simulink model "simulation_environment_thesis"  (R2024b)
settings: Solver=VariableStepAuto  StartTime=0.0  StopTime=3200  RelTol=1e-3
values from neighbouring files (symbols this model uses):
  A = [1, dt; 0, 1]    [estimate_and_control_fully_actuated_with_current.m]
  A1 = [1, 0, 0, 0, dt, 0 ... (first row only)    [estimate_and_control_fully_actuated_with_current.m]
  alpha = 10    [estimate_and_control_fully_actuated_with_current.m]
  alpha_xy_1 = 1    [estimate_and_control_fully_actuated_with_current.m]
  alpha_z_1 = 5    [estimate_and_control_fully_actuated_with_current.m]
  Az1 = [1, dt ... (first row only)    [estimate_and_control_fully_actuated_with_current.m]
  B = [dt; 0]    [estimate_and_control_fully_actuated_with_current.m]
  B1 = [dt, 0 ... (first row only)    [estimate_and_control_fully_actuated_with_current.m]
  Bz1 = [dt ... (first row only)    [estimate_and_control_fully_actuated_with_current.m]
  C1 = [1, 0, 0, 0, 0, 0 ... (first row only)    [estimate_and_control_fully_actuated_with_current.m]
  circumference_x = min_dist_from_ds*cos(theta)    [estimate_and_control_fully_actuated_with_current.m]
  circumference_y = min_dist_from_ds*sin(theta)    [estimate_and_control_fully_actuated_with_current.m]
  const_current = [-r; -s; 0; 0]    [estimate_and_control_fully_actuated_with_current.m]
  csi_psi_1 = 0.0003    [estimate_and_control_fully_actuated_with_current.m]
  csi_r_1 = 0.0003    [estimate_and_control_fully_actuated_with_current.m]
  csi_vcx_1 = 0.0003    [estimate_and_control_fully_actuated_with_current.m]
  csi_vcy_1 = 0.0003    [estimate_and_control_fully_actuated_with_current.m]
  csi_vcz_1 = 0.005    [estimate_and_control_fully_actuated_with_current.m]
  csi_x_1 = 0.003    [estimate_and_control_fully_actuated_with_current.m]
  csi_y_1 = 0.003    [estimate_and_control_fully_actuated_with_current.m]
  csi_z_1 = 0.005    [estimate_and_control_fully_actuated_with_current.m]
  Cz1 = [1, 0]    [estimate_and_control_fully_actuated_with_current.m]
  d = 1    [estimate_and_control_fully_actuated_with_current.m]
  delta_FOV = pi/7    (= 0.448798950513)    [estimate_and_control_fully_actuated_with_current.m]
  desired_speed = speed_0    [estimate_and_control_fully_actuated_with_current.m]
  dt = 1/Tsim    (= 0.005)    [estimate_and_control_fully_actuated_with_current.m]
  eta1 = deg2rad(0.5)    [estimate_and_control_fully_actuated_with_current.m]
  eta2 = deg2rad(0.5)    [estimate_and_control_fully_actuated_with_current.m]
  eta_psi_1 = 0.003    [estimate_and_control_fully_actuated_with_current.m]
  eta_x_1 = 0.03    [estimate_and_control_fully_actuated_with_current.m]
  eta_y_1 = 0.03    [estimate_and_control_fully_actuated_with_current.m]
  eta_z_1 = 0.005    [estimate_and_control_fully_actuated_with_current.m]
  G = dt*eye(2)    [estimate_and_control_fully_actuated_with_current.m]
  G1 = dt*eye(6)    [estimate_and_control_fully_actuated_with_current.m]
  Gz1 = dt*eye(2)    [estimate_and_control_fully_actuated_with_current.m]
  left_reference = L_x*[cos(NED_psi_ds); sin(NED_psi_ds); 0] + L_y_l*[-sin(NED_psi_ds); cos(NED_psi_ds); 0]    [estimate_and_control_fully_actuated_with_current.m]
  max_dist_from_ship = 60    [estimate_and_control_fully_actuated_with_current.m]
  min_dist_from_ds = 45    [estimate_and_control_fully_actuated_with_current.m]
  mu = -5    [estimate_and_control_fully_actuated_with_current.m]
  NED_psi_ds = -pi/2    (= -1.57079632679)    [estimate_and_control_fully_actuated_with_current.m]
  p_b1 = [-90; 90; 5]    [estimate_and_control_fully_actuated_with_current.m]
  position_t_0 = [0; 0; 0; 0]    [estimate_and_control_fully_actuated_with_current.m]
  psi_P = linspace(0,2*pi,360)'    [estimate_and_control_fully_actuated_with_current.m]
  Q = [csi1_kf^2, 0 ... (first row only)    [estimate_and_control_fully_actuated_with_current.m]
  Q1 = [csi_x_1_kf^2, 0, 0, 0, 0, 0 ... (first row only)    [estimate_and_control_fully_actuated_with_current.m]
  Qz1 = [csi_z_1_kf^2, 0 ... (first row only)    [estimate_and_control_fully_actuated_with_current.m]
  R = [eta1_kf^2, 0 ... (first row only)    [estimate_and_control_fully_actuated_with_current.m]
  R1 = [eta_x_1_kf^2, 0, 0 ... (first row only)    [estimate_and_control_fully_actuated_with_current.m]
  right_reference = L_x*[cos(NED_psi_ds); sin(NED_psi_ds); 0] + L_y_r*[sin(NED_psi_ds); -cos(NED_psi_ds); 0]    [estimate_and_control_fully_actuated_with_current.m]
  Rz1 = eta_z_1_kf^2    (= 1e-06)    [estimate_and_control_fully_actuated_with_current.m]
  speed_0 = [0.1, 0.1, 0, 0]'    [estimate_and_control_fully_actuated_with_current.m]
  tau_heave_sat = 30    [estimate_and_control_fully_actuated_with_current.m]
  tau_surge_sat = 10    [estimate_and_control_fully_actuated_with_current.m]
  tau_sway_sat = 30    [estimate_and_control_fully_actuated_with_current.m]
  tau_yaw_sat = 0.1    [estimate_and_control_fully_actuated_with_current.m]
  var_vm_x = 0.001    [estimate_and_control_fully_actuated_with_current.m]
  var_vm_y = 0.001    [estimate_and_control_fully_actuated_with_current.m]
  var_vm_z = 0.001    [estimate_and_control_fully_actuated_with_current.m]
undefined symbols (not defined in the file or in its folder): A2, alpha2, B2, csi1_2, csi2_2, csi3_2, csi4_2, csi5_2, csi6_2, csi7_2, csi8_2, eta1_2, eta2_2, eta3_2, eta4_2, eta5_2, eta6_2, G2, Q2, R2
libraries: simulink x60, simulink_extras x5
(part 6 of 16)

== system simulation_environment_thesis/PHASE 3&4.21/PHASE  3 ==
  note: ds_psi_auv
  note: ok, trasformazione coerente
  note: ok cominciano esattamente dalla flag
  note: physical data to be sent to
      | the range-measurement simulator
  ship_psi_auv : Inport
  ship_p_auv : Inport  Port=2
  ship_p_ds : Inport  Port=3
  Enable : EnablePort
  CONTROLLER4 : Subsystem
  Constant : Constant  Value=0
  Constant9 : Constant  Value=desired_speed
  Data Store Read : DataStoreRead  DataStoreName=x_hat_23_2  IndexOptions=Index vector (dialog)  Indices=1  OutputSizes=1
  Data Store Read1 : DataStoreRead  DataStoreName=P0_23_2  IndexOptions=Index vector (dialog)  Indices=1  OutputSizes=1
  Data Store Write : DataStoreWrite  DataStoreName=x_hat_34_2  IndexOptions=Index vector (dialog)  Indices=1  OutputSizes=1
  Data Store Write1 : DataStoreWrite  DataStoreName=P0_34_2  IndexOptions=Index vector (dialog)  Indices=1  OutputSizes=1
  MATLAB Function : MATLAB Function  TreatAsAtomicUnit=on
  MATLAB Function1 : MATLAB Function  TreatAsAtomicUnit=on
  Scope1 : Scope
  Selector1 : Selector  InputPortWidth=4  IndexOptions=Index vector (dialog)  Indices=[1 2 3]  OutputSizes=1
  Selector2 : Selector  InputPortWidth=3  IndexOptions=Index vector (dialog)  Indices=[3]  OutputSizes=1
  Selector3 : Selector  InputPortWidth=3  IndexOptions=Index vector (dialog)  Indices=[1 2]  OutputSizes=1
  Selector4 : Selector  InputPortWidth=8  IndexOptions=Index vector (dialog)  Indices=[1 2 7 8 4 5]  OutputSizes=1
  Selector5 : Selector  InputPortWidth=8  IndexOptions=Index vector (dialog)  Indices=[3 6]  OutputSizes=1
  Sum : Sum  Inputs=|+-
  TAU : Scope
  To Workspace : ToWorkspace  VariableName=switchposition23  SampleTime=-1
  XY PATH GUIDANCE : Subsystem
  dynamic + kinematic models1 : Subsystem
  "p, v_c, psi, r (3D) estimate, from RANGE, v_c, PSI measurement" : Subsystem
  v error (robot frame)5 : Scope
  yaw_err3 : Scope
  z_err : Scope
  speed body : Outport
  s_p_auv : Outport  Port=2
  s_psi_auv : Outport  Port=3
  ds_p_auv : Outport  Port=4
  state_est_plane : Outport  Port=5
  state_est_z : Outport  Port=6
  "z_err " : Outport  Port=7
  error speed : Outport  Port=8
  yaw_error : Outport  Port=9
  wires:
  CONTROLLER4/1(tau) -> dynamic + kinematic models1/1(tau), TAU/1
  Constant9/1 -> dynamic + kinematic models1/2(ref speed), CONTROLLER4/3(speed_0), XY PATH GUIDANCE/1(speed)
  dynamic + kinematic models1/1(error speed) -> CONTROLLER4/1(v_err), error speed/1, v error (robot frame)5/1
  MATLAB Function/1(ds_p_auv) -> dynamic + kinematic models1/5(ds_p_auv), Scope1/1
  ship_psi_auv/1 -> dynamic + kinematic models1/3(last_yaw__from_ship)
  ship_p_ds/1 -> MATLAB Function1/2(ship_p_ds), MATLAB Function/2(ship_p_ds)
  dynamic + kinematic models1/3(p) -> Selector1/1
  MATLAB Function1/1(ship_p_auv) -> s_p_auv/1
  dynamic + kinematic models1/4(yaw) -> "p, v_c, psi, r (3D) estimate, from RANGE, v_c, PSI measurement"/3(psi), s_psi_auv/1
  dynamic + kinematic models1/5(body speed) -> speed body/1
  Sum/1 -> CONTROLLER4/4(z_err), "z_err "/1, z_err/1
  Constant/1 -> Sum/1
  XY PATH GUIDANCE/1(yaw_error) -> CONTROLLER4/2(psi_err), yaw_err3/1, yaw_error/1
  Selector2/1 -> Sum/2
  "p, v_c, psi, r (3D) estimate, from RANGE, v_c, PSI measurement"/1(xyz_est) -> Selector2/1, Selector3/1
  "p, v_c, psi, r (3D) estimate, from RANGE, v_c, PSI measurement"/2(psi_est) -> dynamic + kinematic models1/4(yaw_est), XY PATH GUIDANCE/3(yaw_est)
  "p, v_c, psi, r (3D) estimate, from RANGE, v_c, PSI measurement"/3(v_c_xyz) -> XY PATH GUIDANCE/4(v_c)
  Selector3/1 -> XY PATH GUIDANCE/2(eta)
  dynamic + kinematic models1/2(v_tot ds) -> "p, v_c, psi, r (3D) estimate, from RANGE, v_c, PSI measurement"/2(inert_vel)
  Selector1/1 -> MATLAB Function1/1(ds_p_auv), ds_p_auv/1, "p, v_c, psi, r (3D) estimate, from RANGE, v_c, PSI measurement"/1(p)
  Data Store Read/1 -> "p, v_c, psi, r (3D) estimate, from RANGE, v_c, PSI measurement"/4(x_0_hat)
  Data Store Read1/1 -> "p, v_c, psi, r (3D) estimate, from RANGE, v_c, PSI measurement"/5(P0)
  "p, v_c, psi, r (3D) estimate, from RANGE, v_c, PSI measurement"/5(P0_out) -> Data Store Write1/1
  ship_p_auv/1 -> MATLAB Function/1(ship_p_auv), To Workspace/1
  Selector5/1 -> state_est_z/1
  "p, v_c, psi, r (3D) estimate, from RANGE, v_c, PSI measurement"/4(state_est) -> Selector5/1, Selector4/1, Data Store Write/1
  Selector4/1 -> state_est_plane/1

== system simulation_environment_thesis/PHASE 3&4.21/PHASE  4 ==
  note: ds_psi_auv
  note: ok, trasformazione coerente
  note: ok cominciano esattamente dalla flag
  note: i know the beacon is my new
      | reference frame, so (0,0,0)
  note: physical data to be sent to
      | the range-measurement simulator
  ship_psi_auv : Inport
  ship_p_auv : Inport  Port=2
  ship_p_ds : Inport  Port=3
  direct_to_4 : Inport  Port=4
  Enable : EnablePort
  CONTROLLER4 : Subsystem
  Constant : Constant  Value=0
  Constant3 : Constant  Value=norm(speed_0)
  Constant5 : Constant  Value=[0; 0]  VectorParams1D=off
  Constant7 : Constant  Value=NED_psi_ds + pi
  Data Store Read : DataStoreRead  DataStoreName=x_hat_23_2  IndexOptions=Index vector (dialog)  Indices=1  OutputSizes=1
  Data Store Read1 : DataStoreRead  DataStoreName=x_hat_34_2  IndexOptions=Index vector (dialog)  Indices=1  OutputSizes=1
  Data Store Read2 : DataStoreRead  DataStoreName=P0_23_2  IndexOptions=Index vector (dialog)  Indices=1  OutputSizes=1
  Data Store Read3 : DataStoreRead  DataStoreName=P0_34_2  IndexOptions=Index vector (dialog)  Indices=1  OutputSizes=1
  MATLAB Function : MATLAB Function  TreatAsAtomicUnit=on
  MATLAB Function1 : MATLAB Function  TreatAsAtomicUnit=on
  MATLAB Function11 : MATLAB Function  TreatAsAtomicUnit=on
  MATLAB Function6 : MATLAB Function  TreatAsAtomicUnit=on
  MATLAB Function7 : MATLAB Function  TreatAsAtomicUnit=on
  MATLAB Function8 : MATLAB Function  Commented=on  TreatAsAtomicUnit=on
  MATLAB Function9 : MATLAB Function  TreatAsAtomicUnit=on
  Memory : Memory
  Scope{1,4,6,8..9} (5 alike) : Scope
  Selector1 : Selector  InputPortWidth=4  IndexOptions=Index vector (dialog)  Indices=[1 2 3]  OutputSizes=1
  Selector3 : Selector  InputPortWidth=3  IndexOptions=Index vector (dialog)  Indices=[3]  OutputSizes=1
  Selector4 : Selector  InputPortWidth=3  IndexOptions=Index vector (dialog)  Indices=[1 2]  OutputSizes=1
  Selector5 : Selector  InputPortWidth=3  IndexOptions=Index vector (dialog)  Indices=[1 2]  OutputSizes=1
  Selector6 : Selector  InputPortWidth=8  IndexOptions=Index vector (dialog)  Indices=[1 2 7 8 4 5]  OutputSizes=1
  Selector7 : Selector  InputPortWidth=8  IndexOptions=Index vector (dialog)  Indices=[3 6]  OutputSizes=1
  Stop Simulation : Stop
  Subsystem : Subsystem
  Sum : Sum  Inputs=|+-
  Sum4 : Sum  Inputs=|+-
  Switch3 : Switch  Criteria=u2 > Threshold  SaturateOnIntegerOverflow=off  InputSameDT=off
  Switch4 : Switch  Criteria=u2 > Threshold  SaturateOnIntegerOverflow=off  InputSameDT=off
  TAU : Scope
  To Workspace : ToWorkspace  VariableName=switchposition34  SampleTime=-1
  To Workspace1 : ToWorkspace  VariableName=docked  SampleTime=-1
  Unit Delay : UnitDelay  SampleTime=-1  HasFrameUpgradeWarning=on
  dynamic + kinematic models1 : Subsystem
  "p, v_c, psi, r (3D) estimate, from RANGE, v_c, PSI measurement" : Subsystem
  "positon and current estimate, XY PLANE from X, Y, psi measurement" : Subsystem  Commented=on
  v error (robot frame)5 : Scope
  z_err : Scope
  speed body : Outport
  s_p_auv : Outport  Port=2
  s_psi_auv : Outport  Port=3
  new_speed_desired : Outport  Port=4
  docked : Outport  Port=5
  state_est_plane : Outport  Port=6
  state_est_z : Outport  Port=7
  ds_p_auv_4 : Outport  Port=8
  z_ err : Outport  Port=9
  angle : Outport  Port=10
  error speed : Outport  Port=11
  wires:
  CONTROLLER4/1(tau) -> dynamic + kinematic models1/1(tau), TAU/1
  dynamic + kinematic models1/1(error speed) -> error speed/1, CONTROLLER4/1(v_err), v error (robot frame)5/1
  MATLAB Function/1(ds_p_auv) -> Scope1/1, dynamic + kinematic models1/5(ds_p_auv)
  ship_psi_auv/1 -> dynamic + kinematic models1/3(last_yaw__from_ship)
  ship_p_ds/1 -> MATLAB Function/2(ship_p_ds), MATLAB Function1/2(ship_p_ds)
  dynamic + kinematic models1/3(p) -> Selector1/1
  MATLAB Function1/1(ship_p_auv) -> s_p_auv/1
  dynamic + kinematic models1/4(yaw) -> s_psi_auv/1, "p, v_c, psi, r (3D) estimate, from RANGE, v_c, PSI measurement"/3(psi)
  dynamic + kinematic models1/5(body speed) -> speed body/1
  Selector3/1 -> Sum/2
  Sum/1 -> z_ err/1, CONTROLLER4/4(z_err), z_err/1
  Constant/1 -> Sum/1
  dynamic + kinematic models1/2(v_tot ds) -> "p, v_c, psi, r (3D) estimate, from RANGE, v_c, PSI measurement"/2(inert_vel)
  Sum4/1 -> MATLAB Function6/1("angle ")
  MATLAB Function6/1(angle) -> angle/1, Scope9/1, CONTROLLER4/2(psi_err)
  Unit Delay/1 -> CONTROLLER4/3(speed_0), dynamic + kinematic models1/2(ref speed)
  "p, v_c, psi, r (3D) estimate, from RANGE, v_c, PSI measurement"/2(psi_est) -> Sum4/2, dynamic + kinematic models1/4(yaw_est)
  Selector1/1 -> "p, v_c, psi, r (3D) estimate, from RANGE, v_c, PSI measurement"/1(p), MATLAB Function1/1(ds_p_auv), ds_p_auv_4/1
  Switch3/1 -> "p, v_c, psi, r (3D) estimate, from RANGE, v_c, PSI measurement"/4(x_0_hat)
  Data Store Read/1 -> Switch3/1
  Data Store Read1/1 -> Switch3/3
  Data Store Read2/1 -> Switch4/1
  Data Store Read3/1 -> Switch4/3
  direct_to_4/1 -> Switch4/2, Switch3/2
  Switch4/1 -> "p, v_c, psi, r (3D) estimate, from RANGE, v_c, PSI measurement"/5(P0)
  ship_p_auv/1 -> To Workspace/1, MATLAB Function/1(ship_p_auv)
  Subsystem/1(w) -> MATLAB Function7/3(w)
  MATLAB Function11/1(angle) -> Subsystem/1(NED_psi_dock), MATLAB Function7/4(NED_psi_dock)
  "p, v_c, psi, r (3D) estimate, from RANGE, v_c, PSI measurement"/3(v_c_xyz) -> Selector5/1
  MATLAB Function7/1(v_blend) -> Scope4/1, Unit Delay/1, new_speed_desired/1, Scope6/1
  "p, v_c, psi, r (3D) estimate, from RANGE, v_c, PSI measurement"/1(xyz_est) -> Selector4/1, Subsystem/3(eta), Selector3/1
  Selector4/1 -> MATLAB Function9/1(ds_p_auv), Subsystem/2(x_y)
  Subsystem/2(speed_desired_circ) -> MATLAB Function7/2(v_circ)
  MATLAB Function9/2(range) -> Scope8/1
  Constant7/1 -> Sum4/1, MATLAB Function11/1("angle ")
  Memory/1 -> MATLAB Function9/3("docked ")
  Subsystem/4(speed_desired_lin) -> MATLAB Function7/1(v_line)
  Constant5/1 -> MATLAB Function9/2(ds_p_ds)
  Constant3/1 -> Subsystem/5(v_tot_des)
  Subsystem/3(d) -> MATLAB Function7/5(d)
  Selector5/1 -> Subsystem/4(v_c)
  "p, v_c, psi, r (3D) estimate, from RANGE, v_c, PSI measurement"/4(state_est) -> Selector6/1, Selector7/1
  Selector7/1 -> state_est_z/1
  Selector6/1 -> state_est_plane/1
  MATLAB Function9/1(docked) -> To Workspace1/1, docked/1, Memory/1, Stop Simulation/1

== system simulation_environment_thesis/PHASE 3&4.21/Subsystem3 ==
  num : Inport
  Enable : EnablePort
  MATLAB Function7 : MATLAB Function  TreatAsAtomicUnit=on
  out : Outport
  wires:
  num/1 -> MATLAB Function7/1(num)
  MATLAB Function7/1(out) -> out/1

== system simulation_environment_thesis/PHASE 3&4.21/end phase 3 ==
  note: the DS is in the origin
      | of the DS frame
  ds_p_auv : Inport
  Enable : EnablePort
  # of pings : MATLAB Function  TreatAsAtomicUnit=on
  Constant : Constant  Value=NED_psi_ds
  Constant13 : Constant  Value=[0; 0]  VectorParams1D=off
  Constant8 : Constant  Value=delta_FOV
  Scope3 : Scope
  num_of_pings : Outport
  wires:
  Constant8/1 -> # of pings/2(delta)
  ds_p_auv/1 -> # of pings/4(ds_p_auv)
  # of pings/1(num_of_pings) -> num_of_pings/1, Scope3/1
  Constant13/1 -> # of pings/3(ds_p_ds)
  Constant/1 -> # of pings/1(alpha_ds)

== system simulation_environment_thesis/PHASE 3&4.21/logic to recognize where i am, based on the # of pings ==
  note: se out è 1 --> phase 4
      | se out è 0 --> phase 3
  num : Inport
  MATLAB Function6 : MATLAB Function  TreatAsAtomicUnit=on
  out : Outport
  wires:
  MATLAB Function6/1(out) -> out/1
  num/1 -> MATLAB Function6/1(num)

== system simulation_environment_thesis/PHASE 3&4.21/simulator of pings from sensor ==
  note: the DS is in the origin
      | of the DS frame
  ds_p_auv : Inport
  stop_flag : Inport  Port=2
  # of pings : MATLAB Function  TreatAsAtomicUnit=on
  Constant : Constant  Value=NED_psi_ds
  Constant13 : Constant  Value=[0; 0]  VectorParams1D=off
  Constant8 : Constant  Value=delta_FOV
  Scope3 : Scope
  # OF PINGS : Outport
  wires:
  Constant/1 -> # of pings/1(alpha_ds)
  # of pings/1(num_of_pings) -> Scope3/1, # OF PINGS/1
  Constant13/1 -> # of pings/3(ds_p_ds)
  Constant8/1 -> # of pings/2(delta)
  ds_p_auv/1 -> # of pings/4(ds_p_auv)
  stop_flag/1 -> # of pings/5(stop_flag)

== system simulation_environment_thesis/PHASE 3&4.22/PHASE  3 ==
  note: ds_psi_auv
  note: ok, trasformazione coerente
  note: ok cominciano esattamente dalla flag
  note: physical data to be sent to
      | the range-measurement simulator
  ship_psi_auv : Inport
  ship_p_auv : Inport  Port=2
  ship_p_ds : Inport  Port=3
  Enable : EnablePort
  CONTROLLER4 : Subsystem
  Constant : Constant  Value=0
  Constant9 : Constant  Value=desired_speed
  Data Store Read : DataStoreRead  DataStoreName=x_hat_23_3  IndexOptions=Index vector (dialog)  Indices=1  OutputSizes=1
  Data Store Read1 : DataStoreRead  DataStoreName=P0_23_3  IndexOptions=Index vector (dialog)  Indices=1  OutputSizes=1
  Data Store Write : DataStoreWrite  DataStoreName=x_hat_34_3  IndexOptions=Index vector (dialog)  Indices=1  OutputSizes=1
  Data Store Write1 : DataStoreWrite  DataStoreName=P0_34_3  IndexOptions=Index vector (dialog)  Indices=1  OutputSizes=1
  MATLAB Function : MATLAB Function  TreatAsAtomicUnit=on
  MATLAB Function1 : MATLAB Function  TreatAsAtomicUnit=on
  Scope1 : Scope
  Selector1 : Selector  InputPortWidth=4  IndexOptions=Index vector (dialog)  Indices=[1 2 3]  OutputSizes=1
  Selector2 : Selector  InputPortWidth=3  IndexOptions=Index vector (dialog)  Indices=[3]  OutputSizes=1
  Selector3 : Selector  InputPortWidth=3  IndexOptions=Index vector (dialog)  Indices=[1 2]  OutputSizes=1
  Selector4 : Selector  InputPortWidth=8  IndexOptions=Index vector (dialog)  Indices=[1 2 7 8 4 5]  OutputSizes=1
  Selector5 : Selector  InputPortWidth=8  IndexOptions=Index vector (dialog)  Indices=[3 6]  OutputSizes=1
  Sum : Sum  Inputs=|+-
  TAU : Scope
  To Workspace : ToWorkspace  VariableName=switchposition23  SampleTime=-1
  XY PATH GUIDANCE : Subsystem
  dynamic + kinematic models1 : Subsystem
  "p, v_c, psi, r (3D) estimate, from RANGE, v_c, PSI measurement" : Subsystem
  v error (robot frame)5 : Scope
  yaw_err3 : Scope
  z_err : Scope
  speed body : Outport
  s_p_auv : Outport  Port=2
  s_psi_auv : Outport  Port=3
  ds_p_auv : Outport  Port=4
  state_est_plane : Outport  Port=5
  state_est_z : Outport  Port=6
  error speed : Outport  Port=7
  yaw_error : Outport  Port=8
  z_ err : Outport  Port=9
  wires:
  Constant9/1 -> dynamic + kinematic models1/2(ref speed), CONTROLLER4/3(speed_0), XY PATH GUIDANCE/1(speed)
  CONTROLLER4/1(tau) -> dynamic + kinematic models1/1(tau), TAU/1
  dynamic + kinematic models1/1(error speed) -> error speed/1, CONTROLLER4/1(v_err), v error (robot frame)5/1
  MATLAB Function/1(ds_p_auv) -> dynamic + kinematic models1/5(ds_p_auv), Scope1/1
  ship_psi_auv/1 -> dynamic + kinematic models1/3(last_yaw__from_ship)
  ship_p_ds/1 -> MATLAB Function1/2(ship_p_ds), MATLAB Function/2(ship_p_ds)
  dynamic + kinematic models1/4(p) -> Selector1/1
  MATLAB Function1/1(ship_p_auv) -> s_p_auv/1
  dynamic + kinematic models1/5(yaw) -> s_psi_auv/1, "p, v_c, psi, r (3D) estimate, from RANGE, v_c, PSI measurement"/3(psi)
  dynamic + kinematic models1/3(body speed) -> speed body/1
  Sum/1 -> CONTROLLER4/4(z_err), z_ err/1, z_err/1
  Constant/1 -> Sum/1
  XY PATH GUIDANCE/1(yaw_error) -> CONTROLLER4/2(psi_err), yaw_err3/1, yaw_error/1
  Selector2/1 -> Sum/2
  "p, v_c, psi, r (3D) estimate, from RANGE, v_c, PSI measurement"/1(xyz_est) -> Selector2/1, Selector3/1
  "p, v_c, psi, r (3D) estimate, from RANGE, v_c, PSI measurement"/2(psi_est) -> dynamic + kinematic models1/4(yaw_est), XY PATH GUIDANCE/3(yaw_est)
  "p, v_c, psi, r (3D) estimate, from RANGE, v_c, PSI measurement"/3(v_c_xyz) -> XY PATH GUIDANCE/4(v_c)
  Selector3/1 -> XY PATH GUIDANCE/2(eta)
  dynamic + kinematic models1/2(v_tot ds) -> "p, v_c, psi, r (3D) estimate, from RANGE, v_c, PSI measurement"/2(inert_vel)
  Selector1/1 -> MATLAB Function1/1(ds_p_auv), ds_p_auv/1, "p, v_c, psi, r (3D) estimate, from RANGE, v_c, PSI measurement"/1(p)
  Data Store Read/1 -> "p, v_c, psi, r (3D) estimate, from RANGE, v_c, PSI measurement"/4(x_0_hat)
  Data Store Read1/1 -> "p, v_c, psi, r (3D) estimate, from RANGE, v_c, PSI measurement"/5(P0)
  "p, v_c, psi, r (3D) estimate, from RANGE, v_c, PSI measurement"/5(P0_out) -> Data Store Write1/1
  ship_p_auv/1 -> MATLAB Function/1(ship_p_auv), To Workspace/1
  Selector5/1 -> state_est_z/1
  Selector4/1 -> state_est_plane/1
  "p, v_c, psi, r (3D) estimate, from RANGE, v_c, PSI measurement"/4(state_est) -> Selector4/1, Selector5/1, Data Store Write/1

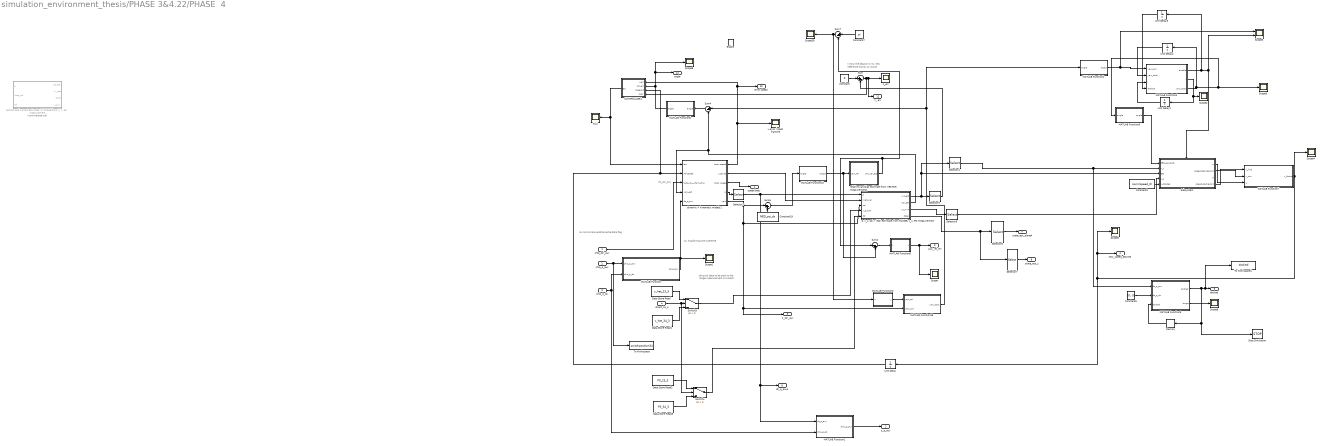
== system simulation_environment_thesis/PHASE 3&4.22/PHASE  4 ==
  note: ds_psi_auv
  note: ok, trasformazione coerente
  note: ok cominciano esattamente dalla flag
  note: i know the beacon is my new
      | reference frame, so (0,0,0)
  note: physical data to be sent to
      | the range-measurement simulator
  ship_psi_auv : Inport
  ship_p_auv : Inport  Port=2
  ship_p_ds : Inport  Port=3
  direct_to_4 : Inport  Port=4
  Enable : EnablePort
  CONTROLLER4 : Subsystem
  Constant : Constant  Value=0
  Constant19 : Constant  Value=NED_psi_ds
  Constant3 : Constant  Value=norm(speed_0)
  Constant5 : Constant  Value=[0; 0]  VectorParams1D=off
  Constant7 : Constant  Value=pi
  Data Store Read : DataStoreRead  DataStoreName=x_hat_23_3  IndexOptions=Index vector (dialog)  Indices=1  OutputSizes=1
  Data Store Read1 : DataStoreRead  DataStoreName=x_hat_34_3  IndexOptions=Index vector (dialog)  Indices=1  OutputSizes=1
  Data Store Read2 : DataStoreRead  DataStoreName=P0_23_3  IndexOptions=Index vector (dialog)  Indices=1  OutputSizes=1
  Data Store Read3 : DataStoreRead  DataStoreName=P0_34_3  IndexOptions=Index vector (dialog)  Indices=1  OutputSizes=1
  MATLAB Function : MATLAB Function  TreatAsAtomicUnit=on
  MATLAB Function1 : MATLAB Function  TreatAsAtomicUnit=on
  MATLAB Function10 : MATLAB Function  TreatAsAtomicUnit=on
  MATLAB Function18 : MATLAB Function  TreatAsAtomicUnit=on
  MATLAB Function2 : MATLAB Function  TreatAsAtomicUnit=on
  MATLAB Function3 : MATLAB Function  TreatAsAtomicUnit=on
  MATLAB Function4 : MATLAB Function  TreatAsAtomicUnit=on
  MATLAB Function5 : MATLAB Function  TreatAsAtomicUnit=on
  MATLAB Function6 : MATLAB Function  TreatAsAtomicUnit=on
  MATLAB Function7 : MATLAB Function  TreatAsAtomicUnit=on
  MATLAB Function8 : MATLAB Function  TreatAsAtomicUnit=on
  MATLAB Function9 : MATLAB Function  TreatAsAtomicUnit=on
  Memory : Memory
  Scope{,1..4,6,8..9,29} (9 alike) : Scope
  Scope5 : Scope  NumInputPorts=2
  Selector1 : Selector  InputPortWidth=4  IndexOptions=Index vector (dialog)  Indices=[1 2 3]  OutputSizes=1
  Selector3 : Selector  InputPortWidth=3  IndexOptions=Index vector (dialog)  Indices=[3]  OutputSizes=1
  Selector4 : Selector  InputPortWidth=3  IndexOptions=Index vector (dialog)  Indices=[1 2]  OutputSizes=1
  Selector5 : Selector  InputPortWidth=3  IndexOptions=Index vector (dialog)  Indices=[1 2]  OutputSizes=1
  Selector6 : Selector  InputPortWidth=8  IndexOptions=Index vector (dialog)  Indices=[1 2 7 8 4 5]  OutputSizes=1
  Selector7 : Selector  InputPortWidth=8  IndexOptions=Index vector (dialog)  Indices=[3 6]  OutputSizes=1
  Stop Simulation : Stop
  Subsystem : Subsystem
  Sum : Sum  Inputs=|+-
  Sum2 : Sum  Inputs=|-+
  Sum4 : Sum  Inputs=|+-
  Sum6 : Sum  Inputs=|-+
  Sum7 : Sum  Inputs=|+-
  Switch3 : Switch  Criteria=u2 > Threshold  SaturateOnIntegerOverflow=off  InputSameDT=off
  Switch4 : Switch  Criteria=u2 > Threshold  SaturateOnIntegerOverflow=off  InputSameDT=off
  TAU : Scope
  To Workspace : ToWorkspace  VariableName=switchposition34  SampleTime=-1
  To Workspace1 : ToWorkspace  VariableName=docked  SampleTime=-1
  Unit Delay{,1..3} (4 alike) : UnitDelay  SampleTime=-1  HasFrameUpgradeWarning=on
  dynamic + kinematic models1 : Subsystem
  "p, v_c, psi, r (3D) estimate, from RANGE, v_c, PSI measurement" : Subsystem
  "positon and current estimate, XY PLANE from X, Y, psi measurement" : Subsystem  Commented=on
  relative yaw/bias estimate from VECTOR measurement1 : Subsystem
  v error (robot frame)5 : Scope
  z_err : Scope
  speed body : Outport
  s_p_auv : Outport  Port=2
  s_psi_auv : Outport  Port=3
  new_speed_desired : Outport  Port=4
  docked : Outport  Port=5
  ds_p_auv4 : Outport  Port=6
  state_est_plane4 : Outport  Port=7
  state_est_z : Outport  Port=8
  yaw_rel_err : Outport  Port=9
  error speed : Outport  Port=10
  angle : Outport  Port=11
  "z_ err " : Outport  Port=12
  wires:
  CONTROLLER4/1(tau) -> dynamic + kinematic models1/1(tau), TAU/1
  dynamic + kinematic models1/1(error speed) -> error speed/1, CONTROLLER4/1(v_err), v error (robot frame)5/1
  MATLAB Function/1(ds_p_auv) -> dynamic + kinematic models1/5(ds_p_auv), Scope1/1
  ship_psi_auv/1 -> dynamic + kinematic models1/3(last_yaw__from_ship)
  ship_p_ds/1 -> MATLAB Function/2(ship_p_ds), MATLAB Function1/2(ship_p_ds)
  dynamic + kinematic models1/4(p) -> Selector1/1
  MATLAB Function1/1(ship_p_auv) -> s_p_auv/1
  dynamic + kinematic models1/5(yaw) -> MATLAB Function18/2(yaw_curr), s_psi_auv/1, "p, v_c, psi, r (3D) estimate, from RANGE, v_c, PSI measurement"/3(psi), Sum6/1
  dynamic + kinematic models1/3(body speed) -> speed body/1
  Selector3/1 -> Sum/2
  Sum/1 -> CONTROLLER4/4(z_err), "z_ err "/1, z_err/1
  Constant/1 -> Sum/1
  dynamic + kinematic models1/2(v_tot ds) -> "p, v_c, psi, r (3D) estimate, from RANGE, v_c, PSI measurement"/2(inert_vel)
  "p, v_c, psi, r (3D) estimate, from RANGE, v_c, PSI measurement"/3(v_c_xyz) -> Selector5/1
  Sum4/1 -> MATLAB Function6/1("angle ")
  MATLAB Function6/1(angle) -> angle/1, Scope9/1, CONTROLLER4/2(psi_err)
  "p, v_c, psi, r (3D) estimate, from RANGE, v_c, PSI measurement"/2(psi_est) -> dynamic + kinematic models1/4(yaw_est), Sum4/2
  Selector1/1 -> "p, v_c, psi, r (3D) estimate, from RANGE, v_c, PSI measurement"/1(p), ds_p_auv4/1, MATLAB Function1/1(ds_p_auv)
  Switch3/1 -> "p, v_c, psi, r (3D) estimate, from RANGE, v_c, PSI measurement"/4(x_0_hat)
  Data Store Read/1 -> Switch3/1
  Data Store Read1/1 -> Switch3/3
  Data Store Read2/1 -> Switch4/1
  Data Store Read3/1 -> Switch4/3
  direct_to_4/1 -> Switch4/2, Switch3/2
  Switch4/1 -> "p, v_c, psi, r (3D) estimate, from RANGE, v_c, PSI measurement"/5(P0)
  Constant19/1 -> Sum6/2
  Sum6/1 -> MATLAB Function10/1("angle ")
  Constant7/1 -> Sum7/1
  relative yaw/bias estimate from VECTOR measurement1/1(yaw_rel_est) -> Sum2/1, Sum7/2
  Sum7/1 -> Scope29/1, MATLAB Function3/1(u)
  Unit Delay/1 -> dynamic + kinematic models1/2(ref speed), CONTROLLER4/3(speed_0)
  ship_p_auv/1 -> MATLAB Function/1(ship_p_auv), To Workspace/1
  Constant5/1 -> MATLAB Function9/2(ds_p_ds)
  MATLAB Function7/1(v_blend) -> Scope4/1, Unit Delay/1, Scope6/1, new_speed_desired/1
  "p, v_c, psi, r (3D) estimate, from RANGE, v_c, PSI measurement"/1(xyz_est) -> Subsystem/3(eta), Selector4/1, Selector3/1
  Subsystem/1(w) -> MATLAB Function7/3(w)
  Subsystem/2(speed_desired_circ) -> MATLAB Function7/2(v_circ)
  Selector5/1 -> Subsystem/4(v_c)
  Subsystem/4(speed_desired_lin) -> MATLAB Function7/1(v_line)
  Selector4/1 -> MATLAB Function9/1(ds_p_auv), Subsystem/2(x_y)
  MATLAB Function9/2(range) -> Scope8/1
  Constant3/1 -> Subsystem/5(v_tot_des)
  MATLAB Function18/1(yaw_dock) -> MATLAB Function2/1("angle "), Sum4/1
  Memory/1 -> MATLAB Function9/3("docked ")
  Selector7/1 -> state_est_z/1
  Selector6/1 -> state_est_plane4/1
  "p, v_c, psi, r (3D) estimate, from RANGE, v_c, PSI measurement"/4(state) -> Selector7/1, Selector6/1
  MATLAB Function9/1(docked) -> Stop Simulation/1, Memory/1, To Workspace1/1, docked/1
  MATLAB Function2/1(angle) -> Scope5/1, MATLAB Function4/1(yaw_curr)
  MATLAB Function4/1(enable) -> Unit Delay3/1, Subsystem/Enable, Scope5/2
  MATLAB Function5/1(angle) -> Subsystem/1(NED_psi_dock)
  Unit Delay1/1 -> MATLAB Function4/3("i ")
  MATLAB Function4/2(i) -> Scope2/1, Unit Delay1/1
  Unit Delay2/1 -> MATLAB Function4/2("yaw_dock ")
  Unit Delay3/1 -> MATLAB Function4/4("enable ")
  MATLAB Function4/3(yaw_dock) -> Unit Delay2/1, MATLAB Function5/1("angle "), Scope3/1
  MATLAB Function3/1(y) -> MATLAB Function18/1(yaw_err)
  MATLAB Function10/1(angle) -> Sum2/2, relative yaw/bias estimate from VECTOR measurement1/1(yaw_rel)
  Sum2/1 -> MATLAB Function8/1(u)
  MATLAB Function8/1(y) -> Scope/1, yaw_rel_err/1

== system simulation_environment_thesis/PHASE 3&4.22/Subsystem3 ==
  num : Inport
  Enable : EnablePort
  MATLAB Function7 : MATLAB Function  TreatAsAtomicUnit=on
  out : Outport
  wires:
  num/1 -> MATLAB Function7/1(num)
  MATLAB Function7/1(out) -> out/1

== system simulation_environment_thesis/PHASE 3&4.22/end phase 3 ==
  note: the DS is in the origin
      | of the DS frame
  ds_p_auv : Inport
  Enable : EnablePort
  # of pings : MATLAB Function  TreatAsAtomicUnit=on
  Constant : Constant  Value=NED_psi_ds
  Constant13 : Constant  Value=[0; 0]  VectorParams1D=off
  Constant8 : Constant  Value=delta_FOV
  Scope3 : Scope
  num_of_pings : Outport
  wires:
  Constant8/1 -> # of pings/2(delta)
  ds_p_auv/1 -> # of pings/4(ds_p_auv)
  # of pings/1(num_of_pings) -> num_of_pings/1, Scope3/1
  Constant13/1 -> # of pings/3(ds_p_ds)
  Constant/1 -> # of pings/1(alpha_ds)

== system simulation_environment_thesis/PHASE 3&4.22/logic to recognize where i am, based on the # of pings ==
  note: se out è 1 --> phase 4
      | se out è 0 --> phase 3
  num : Inport
  MATLAB Function6 : MATLAB Function  TreatAsAtomicUnit=on
  out : Outport
  wires:
  MATLAB Function6/1(out) -> out/1
  num/1 -> MATLAB Function6/1(num)

== system simulation_environment_thesis/PHASE 3&4.22/simulator of pings from sensor ==
  note: the DS is in the origin
      | of the DS frame
  ds_p_auv : Inport
  stop_flag : Inport  Port=2
  # of pings : MATLAB Function  TreatAsAtomicUnit=on
  Constant : Constant  Value=NED_psi_ds
  Constant13 : Constant  Value=[0; 0]  VectorParams1D=off
  Constant8 : Constant  Value=delta_FOV
  Scope3 : Scope
  # OF PINGS : Outport
  wires:
  Constant/1 -> # of pings/1(alpha_ds)
  # of pings/1(num_of_pings) -> Scope3/1, # OF PINGS/1
  Constant13/1 -> # of pings/3(ds_p_ds)
  Constant8/1 -> # of pings/2(delta)
  ds_p_auv/1 -> # of pings/4(ds_p_auv)
  stop_flag/1 -> # of pings/5(stop_flag)

== system simulation_environment_thesis/PHASE 3&4.24/PHASE  3 ==
  note: ds_psi_auv
  note: ok, trasformazione coerente
  note: ok cominciano esattamente dalla flag
  ship_psi_auv : Inport
  ship_p_auv : Inport  Port=2
  ship_p_ds : Inport  Port=3
  Enable : EnablePort
  CONTROLLER4 : Subsystem
  Constant : Constant  Value=0
  Constant9 : Constant  Value=desired_speed
  Data Store Read : DataStoreRead  DataStoreName=x_hat_23_z_1  IndexOptions=Index vector (dialog)  Indices=1  OutputSizes=1
  Data Store Read1 : DataStoreRead  DataStoreName=P0_23_z_1  IndexOptions=Index vector (dialog)  Indices=1  OutputSizes=1
  Data Store Read2 : DataStoreRead  DataStoreName=x_hat_23_xy_1  IndexOptions=Index vector (dialog)  Indices=1  OutputSizes=1
  Data Store Read3 : DataStoreRead  DataStoreName=P0_23_xy_1  IndexOptions=Index vector (dialog)  Indices=1  OutputSizes=1
  Data Store Write : DataStoreWrite  DataStoreName=x_hat_34_z_1  IndexOptions=Index vector (dialog)  Indices=1  OutputSizes=1
  Data Store Write1 : DataStoreWrite  DataStoreName=P0_34_z_1  IndexOptions=Index vector (dialog)  Indices=1  OutputSizes=1
  Data Store Write2 : DataStoreWrite  DataStoreName=x_hat_34_xy_1  IndexOptions=Index vector (dialog)  Indices=1  OutputSizes=1
  Data Store Write3 : DataStoreWrite  DataStoreName=P0_34_xy_1  IndexOptions=Index vector (dialog)  Indices=1  OutputSizes=1
  MATLAB Function : MATLAB Function  TreatAsAtomicUnit=on
  MATLAB Function1 : MATLAB Function  TreatAsAtomicUnit=on
  Scope1 : Scope
  Selector1 : Selector  InputPortWidth=4  IndexOptions=Index vector (dialog)  Indices=[1 2 3]  OutputSizes=1
  Sum : Sum  Inputs=|+-
  TAU : Scope
  To Workspace : ToWorkspace  VariableName=switchposition23  SampleTime=-1
  XY PATH GUIDANCE : Subsystem
  dynamic + kinematic models1 : Subsystem
  "positon and current estimate, XY PLANE from X, Y, psi measurement" : Subsystem
  v error (robot frame)5 : Scope
  yaw_err3 : Scope
  "z, v_c_z estimate from DEPTH measurement" : Subsystem
  z_err : Scope
  speed body : Outport
  s_p_auv : Outport  Port=2
  s_psi_auv : Outport  Port=3
  ds_p_auv : Outport  Port=4
  state_est_plane : Outport  Port=5
  state_est_z : Outport  Port=6
  error speed : Outport  Port=7
  yaw_error : Outport  Port=8
  "z_err " : Outport  Port=9
  wires:
  "positon and current estimate, XY PLANE from X, Y, psi measurement"/2(psi_est) -> dynamic + kinematic models1/4(yaw_est), XY PATH GUIDANCE/3(yaw_est)
  Constant9/1 -> dynamic + kinematic models1/2(ref speed), CONTROLLER4/3(speed_0), XY PATH GUIDANCE/1(speed)
  CONTROLLER4/1(tau) -> dynamic + kinematic models1/1(tau), TAU/1
  dynamic + kinematic models1/1(error speed) -> error speed/1, CONTROLLER4/1(v_err), v error (robot frame)5/1
  MATLAB Function/1(ds_p_auv) -> dynamic + kinematic models1/5(ds_p_auv), Scope1/1
  ship_psi_auv/1 -> dynamic + kinematic models1/3(last_yaw__from_ship)
  ship_p_ds/1 -> MATLAB Function1/2(ship_p_ds), MATLAB Function/2(ship_p_ds)
  dynamic + kinematic models1/4(p) -> "positon and current estimate, XY PLANE from X, Y, psi measurement"/1(p), Selector1/1
  Selector1/1 -> "z, v_c_z estimate from DEPTH measurement"/1(p), MATLAB Function1/1(ds_p_auv), ds_p_auv/1
  MATLAB Function1/1(ship_p_auv) -> s_p_auv/1
  dynamic + kinematic models1/5(yaw) -> s_psi_auv/1, "positon and current estimate, XY PLANE from X, Y, psi measurement"/3(psi)
  dynamic + kinematic models1/2(v_tot ds) -> "positon and current estimate, XY PLANE from X, Y, psi measurement"/2(inert_vel)
  dynamic + kinematic models1/3(body speed) -> speed body/1
  "z, v_c_z estimate from DEPTH measurement"/1(z_est) -> Sum/2
  Sum/1 -> "z_err "/1, CONTROLLER4/4(z_err), z_err/1
  Constant/1 -> Sum/1
  "positon and current estimate, XY PLANE from X, Y, psi measurement"/3(v_c_xy) -> XY PATH GUIDANCE/4(v_c)
  XY PATH GUIDANCE/1(yaw_error) -> yaw_error/1, CONTROLLER4/2(psi_err), yaw_err3/1
  "positon and current estimate, XY PLANE from X, Y, psi measurement"/1(xy_est) -> XY PATH GUIDANCE/2(eta)
  Data Store Read/1 -> "z, v_c_z estimate from DEPTH measurement"/2(x_0_hat_z)
  Data Store Read1/1 -> "z, v_c_z estimate from DEPTH measurement"/3(P0_z)
  "z, v_c_z estimate from DEPTH measurement"/2(state_est) -> state_est_z/1, Data Store Write/1
  "z, v_c_z estimate from DEPTH measurement"/3(P0) -> Data Store Write1/1
  Data Store Read2/1 -> "positon and current estimate, XY PLANE from X, Y, psi measurement"/4(x_0_hat_xy)
  Data Store Read3/1 -> "positon and current estimate, XY PLANE from X, Y, psi measurement"/5(P0_xy)
  "positon and current estimate, XY PLANE from X, Y, psi measurement"/4(state_est) -> state_est_plane/1, Data Store Write2/1
  "positon and current estimate, XY PLANE from X, Y, psi measurement"/5(P0) -> Data Store Write3/1
  ship_p_auv/1 -> To Workspace/1, MATLAB Function/1(ship_p_auv)

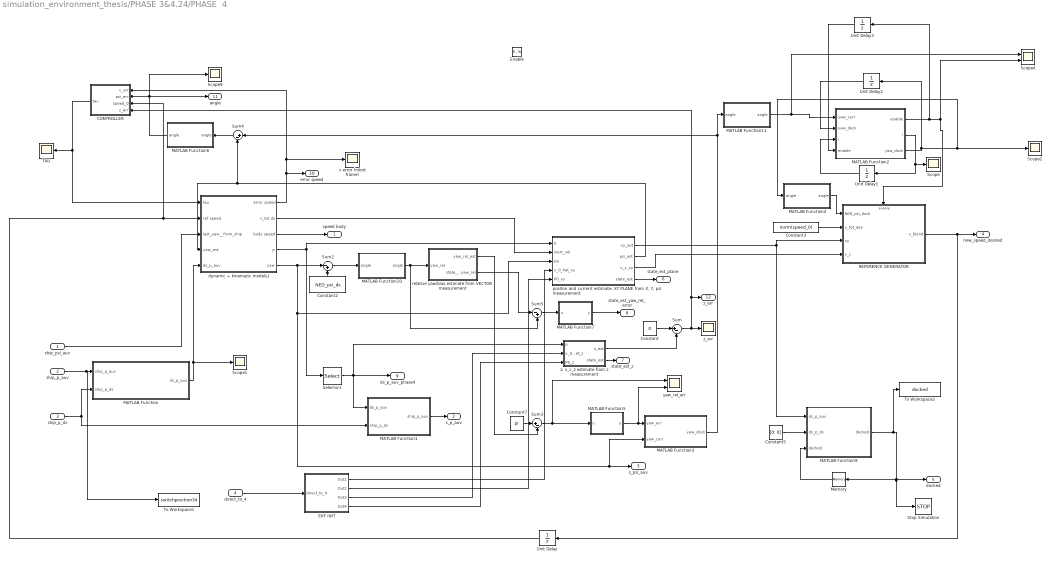
== system simulation_environment_thesis/PHASE 3&4.24/PHASE  4 ==
  ship_psi_auv : Inport
  ship_p_auv : Inport  Port=2
  ship_p_ds : Inport  Port=3
  direct_to_4 : Inport  Port=4
  Enable : EnablePort
  CONTROLLER : Subsystem
  Constant : Constant  Value=0
  Constant2 : Constant  Value=NED_psi_ds
  Constant3 : Constant  Value=norm(speed_0)
  Constant5 : Constant  Value=[0; 0]  VectorParams1D=off
  Constant7 : Constant  Value=pi
  EKF INIT : Subsystem
  MATLAB Function : MATLAB Function  TreatAsAtomicUnit=on
  MATLAB Function1 : MATLAB Function  TreatAsAtomicUnit=on
  MATLAB Function10 : MATLAB Function  TreatAsAtomicUnit=on
  MATLAB Function11 : MATLAB Function  TreatAsAtomicUnit=on
  MATLAB Function2 : MATLAB Function  TreatAsAtomicUnit=on
  MATLAB Function3 : MATLAB Function  TreatAsAtomicUnit=on
  MATLAB Function4 : MATLAB Function  TreatAsAtomicUnit=on
  MATLAB Function5 : MATLAB Function  TreatAsAtomicUnit=on
  MATLAB Function6 : MATLAB Function  TreatAsAtomicUnit=on
  MATLAB Function7 : MATLAB Function  TreatAsAtomicUnit=on
  MATLAB Function9 : MATLAB Function  TreatAsAtomicUnit=on
  Memory : Memory
  REFERENCE GENERATOR : Subsystem
  Scope{,1..2,9} (4 alike) : Scope
  Scope4 : Scope  NumInputPorts=2
  Selector1 : Selector  InputPortWidth=4  IndexOptions=Index vector (dialog)  Indices=[1 2 3]  OutputSizes=1
  Stop Simulation : Stop
  Sum : Sum  Inputs=|+-
  Sum2 : Sum  Inputs=|-+
  Sum3 : Sum  Inputs=|+-
  Sum4 : Sum  Inputs=|+-
  Sum5 : Sum  Inputs=|-+
  TAU : Scope
  To Workspace1 : ToWorkspace  VariableName=switchposition34  SampleTime=-1
  To Workspace2 : ToWorkspace  VariableName=docked  SampleTime=-1
  Unit Delay{,1..3} (4 alike) : UnitDelay  SampleTime=-1  HasFrameUpgradeWarning=on
  dynamic + kinematic models1 : Subsystem
  "positon and current estimate, XY PLANE from X, Y, psi measurement" : Subsystem
  relative yaw/bias estimate from VECTOR measurement : Subsystem
  v error (robot frame) : Scope
  yaw_rel_err : Scope  NumInputPorts=2
  "z, v_c_z estimate from z measurement" : Subsystem
  z_err : Scope
  speed body : Outport
  s_p_auv : Outport  Port=2
  s_psi_auv : Outport  Port=3
  new_speed_desired : Outport  Port=4
  docked : Outport  Port=5
  state_est_plane : Outport  Port=6
  state_est_z : Outport  Port=7
  state_est_yaw_rel_error : Outport  Port=8
  ds_p_auv_phase4 : Outport  Port=9
  error speed : Outport  Port=10
  angle : Outport  Port=11
  "z_err " : Outport  Port=12
  wires:
  CONTROLLER/1(tau) -> dynamic + kinematic models1/1(tau), TAU/1
  dynamic + kinematic models1/1(error speed) -> CONTROLLER/1(v_err), v error (robot frame)/1, error speed/1
  MATLAB Function/1(ds_p_auv) -> dynamic + kinematic models1/5(ds_p_auv), Scope1/1
  ship_psi_auv/1 -> dynamic + kinematic models1/3(last_yaw__from_ship)
  ship_p_ds/1 -> MATLAB Function1/2(ship_p_ds), MATLAB Function/2(ship_p_ds)
  dynamic + kinematic models1/4(p) -> "positon and current estimate, XY PLANE from X, Y, psi measurement"/1(p), Selector1/1
  Selector1/1 -> MATLAB Function1/1(ds_p_auv), ds_p_auv_phase4/1, "z, v_c_z estimate from z measurement"/1(p)
  MATLAB Function1/1(ship_p_auv) -> s_p_auv/1
  dynamic + kinematic models1/5(yaw) -> MATLAB Function3/2(yaw_curr), s_psi_auv/1, "positon and current estimate, XY PLANE from X, Y, psi measurement"/3(psi), Sum2/1
  dynamic + kinematic models1/3(body speed) -> speed body/1
  "z, v_c_z estimate from z measurement"/1(z_est) -> Sum/2
  Sum/1 -> CONTROLLER/4(z_err), "z_err "/1, z_err/1
  Constant/1 -> Sum/1
  relative yaw/bias estimate from VECTOR measurement/1(yaw_rel_est) -> Sum3/2
  Constant2/1 -> Sum2/2
  Sum2/1 -> MATLAB Function10/1("angle ")
  dynamic + kinematic models1/2(v_tot ds) -> "positon and current estimate, XY PLANE from X, Y, psi measurement"/2(inert_vel)
  "positon and current estimate, XY PLANE from X, Y, psi measurement"/2(psi_est) -> dynamic + kinematic models1/4(yaw_est), Sum4/2
  Constant7/1 -> Sum3/1
  Sum3/1 -> yaw_rel_err/1, MATLAB Function5/1(u)
  Sum4/1 -> MATLAB Function6/1("angle ")
  MATLAB Function6/1(angle) -> angle/1, Scope9/1, CONTROLLER/2(psi_err)
  Constant5/1 -> MATLAB Function9/2(ds_p_ds)
  Memory/1 -> MATLAB Function9/3("docked ")
  direct_to_4/1 -> EKF INIT/1(direct_to_4)
  EKF INIT/1(Out1) -> "positon and current estimate, XY PLANE from X, Y, psi measurement"/4(x_0_hat_xy)
  EKF INIT/2(Out2) -> "positon and current estimate, XY PLANE from X, Y, psi measurement"/5(P0_xy)
  EKF INIT/3(Out3) -> "z, v_c_z estimate from z measurement"/2(x_0_hat_z)
  EKF INIT/4(Out4) -> "z, v_c_z estimate from z measurement"/3(P0_z)
  Unit Delay/1 -> dynamic + kinematic models1/2(ref speed), CONTROLLER/3(speed_0)
  ship_p_auv/1 -> To Workspace1/1, MATLAB Function/1(ship_p_auv)
  MATLAB Function3/1(yaw_dock) -> MATLAB Function11/1("angle "), Sum4/1
  MATLAB Function10/1(angle) -> Sum5/2, relative yaw/bias estimate from VECTOR measurement/1(yaw_rel)
  MATLAB Function2/1(enable) -> Unit Delay3/1, Scope4/2, REFERENCE GENERATOR/Enable
  "positon and current estimate, XY PLANE from X, Y, psi measurement"/4(state_est) -> state_est_plane/1
  "z, v_c_z estimate from z measurement"/2(state_est) -> state_est_z/1
  Sum5/1 -> MATLAB Function7/1(u)
  relative yaw/bias estimate from VECTOR measurement/2(state_est_yaw_rel) -> Sum5/1
  ? -> ?
  "positon and current estimate, XY PLANE from X, Y, psi measurement"/1(xy_est) -> MATLAB Function9/1(ds_p_auv), REFERENCE GENERATOR/3(xy)
  REFERENCE GENERATOR/1(v_blend) -> new_speed_desired/1, Unit Delay/1
  Constant3/1 -> REFERENCE GENERATOR/2(v_tot_des)
  "positon and current estimate, XY PLANE from X, Y, psi measurement"/3(v_c_xy) -> REFERENCE GENERATOR/4(v_c)
  MATLAB Function9/1(docked) -> To Workspace2/1, Memory/1, Stop Simulation/1, docked/1
  MATLAB Function11/1(angle) -> Scope4/1, MATLAB Function2/1(yaw_curr)
  MATLAB Function2/3(yaw_dock) -> Unit Delay2/1, MATLAB Function4/1("angle "), Scope2/1
  MATLAB Function4/1(angle) -> REFERENCE GENERATOR/1(NED_psi_dock)
  MATLAB Function5/1(y) -> yaw_rel_err/2, MATLAB Function3/1(yaw_err)
  MATLAB Function2/2(i) -> Scope/1, Unit Delay1/1
  Unit Delay1/1 -> MATLAB Function2/3("i ")
  Unit Delay2/1 -> MATLAB Function2/2("yaw_dock ")
  Unit Delay3/1 -> MATLAB Function2/4("enable ")
  MATLAB Function7/1(y) -> state_est_yaw_rel_error/1

== system simulation_environment_thesis/PHASE 3&4.24/Subsystem3 ==
  num : Inport
  Enable : EnablePort
  MATLAB Function7 : MATLAB Function  TreatAsAtomicUnit=on
  out : Outport
  wires:
  num/1 -> MATLAB Function7/1(num)
  MATLAB Function7/1(out) -> out/1

== system simulation_environment_thesis/PHASE 3&4.24/end phase 3 ==
  note: the DS is in the origin
      | of the DS frame
  ds_p_auv : Inport
  Enable : EnablePort
  # of pings : MATLAB Function  TreatAsAtomicUnit=on
  Constant : Constant  Value=NED_psi_ds
  Constant13 : Constant  Value=[0; 0]  VectorParams1D=off
  Constant8 : Constant  Value=delta_FOV
  Scope3 : Scope
  num_of_pings : Outport
  wires:
  Constant8/1 -> # of pings/2(delta)
  ds_p_auv/1 -> # of pings/4(ds_p_auv)
  # of pings/1(num_of_pings) -> num_of_pings/1, Scope3/1
  Constant13/1 -> # of pings/3(ds_p_ds)
  Constant/1 -> # of pings/1(alpha_ds)

== system simulation_environment_thesis/PHASE 3&4.24/logic to recognize where i am, based on the # of pings ==
  note: se out è 1 --> phase 4
      | se out è 0 --> phase 3
  num : Inport
  MATLAB Function6 : MATLAB Function  TreatAsAtomicUnit=on
  out : Outport
  wires:
  MATLAB Function6/1(out) -> out/1
  num/1 -> MATLAB Function6/1(num)

== system simulation_environment_thesis/PHASE 3&4.24/simulator of pings from sensor ==
  note: the DS is in the origin
      | of the DS frame
  ds_p_auv : Inport
  stop_flag : Inport  Port=2
  # of pings : MATLAB Function  TreatAsAtomicUnit=on
  Constant : Constant  Value=NED_psi_ds
  Constant13 : Constant  Value=[0; 0]  VectorParams1D=off
  Constant8 : Constant  Value=delta_FOV
  Scope3 : Scope
  # OF PINGS : Outport
  wires:
  Constant/1 -> # of pings/1(alpha_ds)
  # of pings/1(num_of_pings) -> Scope3/1, # OF PINGS/1
  Constant13/1 -> # of pings/3(ds_p_ds)
  Constant8/1 -> # of pings/2(delta)
  ds_p_auv/1 -> # of pings/4(ds_p_auv)
  stop_flag/1 -> # of pings/5(stop_flag)

== system simulation_environment_thesis/PHASE 1  /CONTROLLER1/ANTIWINDUP SURGE ==
  in : Inport
  K_int : Inport  Port=2
  K_prop : Inport  Port=3
  K_t_u : Inport  Port=4
  Integrator21 : Integrator
  MATLAB Function21 : MATLAB Function  TreatAsAtomicUnit=on
  MATLAB Function22 : MATLAB Function  TreatAsAtomicUnit=on
  MATLAB Function23 : MATLAB Function  TreatAsAtomicUnit=on
  Saturation2 : Saturate  UpperLimit=tau_surge_sat  LowerLimit=-tau_surge_sat
  Sum11 : Sum  Inputs=|++
  Sum12 : Sum  Inputs=|+-
  Sum13 : Sum  Inputs=|++
  tau_u : Outport
  wires:
  in/1 -> MATLAB Function22/1(in), MATLAB Function21/1(in)
  Sum12/1 -> MATLAB Function23/1(e_s_u)
  Sum11/1 -> Integrator21/1
  Sum13/1 -> Saturation2/1, Sum12/2
  MATLAB Function22/1(y) -> Sum11/1
  MATLAB Function21/1(y) -> Sum13/1
  Saturation2/1 -> tau_u/1, Sum12/1
  K_prop/1 -> MATLAB Function21/2(K)
  K_t_u/1 -> MATLAB Function23/2(K_t_u)
  K_int/1 -> MATLAB Function22/2(K)
  MATLAB Function23/1(y) -> Sum11/2
  Integrator21/1 -> Sum13/2

== system simulation_environment_thesis/PHASE 1  /CONTROLLER1/ANTIWINDUP SWAY ==
  in : Inport
  K_int : Inport  Port=2
  K_prop : Inport  Port=3
  K_t_v : Inport  Port=4
  Integrator21 : Integrator
  MATLAB Function21 : MATLAB Function  TreatAsAtomicUnit=on
  MATLAB Function22 : MATLAB Function  TreatAsAtomicUnit=on
  MATLAB Function23 : MATLAB Function  TreatAsAtomicUnit=on
  Saturation2 : Saturate  UpperLimit=tau_sway_sat  LowerLimit=-tau_sway_sat
  Sum11 : Sum  Inputs=|++
  Sum12 : Sum  Inputs=|+-
  Sum13 : Sum  Inputs=|++
  tau_v : Outport
  wires:
  in/1 -> MATLAB Function22/1(in), MATLAB Function21/1(in)
  Sum12/1 -> MATLAB Function23/1(e_s_v)
  Sum11/1 -> Integrator21/1
  Sum13/1 -> Saturation2/1, Sum12/2
  MATLAB Function22/1(y) -> Sum11/1
  MATLAB Function21/1(y) -> Sum13/1
  Saturation2/1 -> tau_v/1, Sum12/1
  K_prop/1 -> MATLAB Function21/2(K)
  K_t_v/1 -> MATLAB Function23/2(K_t_v)
  K_int/1 -> MATLAB Function22/2(K)
  MATLAB Function23/1(y) -> Sum11/2
  Integrator21/1 -> Sum13/2

== system simulation_environment_thesis/PHASE 1  /CONTROLLER1/ANTIWINDUP_HEAVE ==
  K_int : Inport
  K_prop : Inport  Port=2
  K_t_w : Inport  Port=3
  z_err : Inport  Port=4
  w_err : Inport  Port=5
  K_der : Inport  Port=6
  Integrator2 : Integrator
  MATLAB Function11 : MATLAB Function  TreatAsAtomicUnit=on
  MATLAB Function14 : MATLAB Function  TreatAsAtomicUnit=on
  MATLAB Function15 : MATLAB Function  TreatAsAtomicUnit=on
  MATLAB Function16 : MATLAB Function  TreatAsAtomicUnit=on
  Saturation1 : Saturate  UpperLimit=tau_heave_sat  LowerLimit=-tau_heave_sat
  Sum4 : Sum  Inputs=|+-
  Sum8 : Sum  Inputs=|+++
  Sum9 : Sum  Inputs=|++
  tau_w : Outport
  wires:
  w_err/1 -> MATLAB Function16/1(in)
  MATLAB Function11/1(y) -> Sum9/2
  MATLAB Function14/1(y) -> Sum8/1
  MATLAB Function16/1(y) -> Sum8/3
  K_t_w/1 -> MATLAB Function11/2(K_t_w)
  MATLAB Function15/1(y) -> Sum9/1
  Integrator2/1 -> Sum8/2
  K_der/1 -> MATLAB Function16/2(K)
  K_prop/1 -> MATLAB Function14/2(K)
  K_int/1 -> MATLAB Function15/2(K)
  Saturation1/1 -> tau_w/1, Sum4/1
  Sum4/1 -> MATLAB Function11/1(e_s_w)
  z_err/1 -> MATLAB Function15/1(in), MATLAB Function14/1(in)
  Sum9/1 -> Integrator2/1
  Sum8/1 -> Sum4/2, Saturation1/1

== system simulation_environment_thesis/PHASE 1  /CONTROLLER1/ANTIWINDUP_YAW ==
  K_int : Inport
  K_prop : Inport  Port=2
  K_t_psi : Inport  Port=3
  in1 : Inport  Port=4
  in : Inport  Port=5
  K_der : Inport  Port=6
  Integrator2 : Integrator
  MATLAB Function11 : MATLAB Function  TreatAsAtomicUnit=on
  MATLAB Function14 : MATLAB Function  TreatAsAtomicUnit=on
  MATLAB Function15 : MATLAB Function  TreatAsAtomicUnit=on
  MATLAB Function16 : MATLAB Function  TreatAsAtomicUnit=on
  Saturation1 : Saturate  UpperLimit=tau_yaw_sat  LowerLimit=-tau_yaw_sat
  Sum4 : Sum  Inputs=|+-
  Sum8 : Sum  Inputs=|+++
  Sum9 : Sum  Inputs=|++
  tau_psi : Outport
  wires:
  MATLAB Function15/1(y) -> Sum9/1
  Sum8/1 -> Sum4/2, Saturation1/1
  in1/1 -> MATLAB Function15/1(in), MATLAB Function14/1(in)
  Saturation1/1 -> tau_psi/1, Sum4/1
  Sum9/1 -> Integrator2/1
  MATLAB Function14/1(y) -> Sum8/1
  MATLAB Function11/1(y) -> Sum9/2
  Integrator2/1 -> Sum8/2
  Sum4/1 -> MATLAB Function11/1(e_s_psi)
  K_t_psi/1 -> MATLAB Function11/2(K_t_psi)
  K_int/1 -> MATLAB Function15/2(K)
  K_prop/1 -> MATLAB Function14/2(K)
  MATLAB Function16/1(y) -> Sum8/3
  in/1 -> MATLAB Function16/1(in)
  K_der/1 -> MATLAB Function16/2(K)
